simulink model "wlan_phy_rx_pmd"  (Simulink 7.8)
settings: Solver=VariableStepDiscrete  SolverMode=SingleTasking  StartTime=0.0  StopTime=rx_sim.sim_time  RelTol=1e-3
PostLoadFcn:
  | wlan_phy_rx_init
InitFcn:
  | wlan_phy_rx_init
StartFcn:
  | wlan_phy_rx_init
values from neighbouring files (symbols this model uses):
  barker_seq20 = 0.95 * circshift(barker_seq20, [0 4]) ./ max(abs(barker_seq20))    [wlan_phy_rx_init.m]
  bit_scrambler_lfsr_bytes = sum(bit_scrambler_lfsr_bytes .* repmat(2.^[0:7], 127, 1),2)    [wlan_phy_rx_init.m]
  longCorr_coef_i = [3*real(longCorr_coef)]    [wlan_phy_rx_init.m]
  longCorr_coef_q = [3*imag(longCorr_coef)]    [wlan_phy_rx_init.m]
  MAX_CP_LEN = 32    [wlan_phy_rx_init.m]
  MAX_NCBPS = NCBPS_11n_64QAM    (= 312)    [wlan_phy_rx_init.m]
  MAX_NUM_BYTES = 2^16-1    (= 65535)    [wlan_phy_rx_init.m]
  MAX_NUM_SC = 64    [wlan_phy_rx_init.m]
  MAX_NUM_SYMS = 600    [wlan_phy_rx_init.m]
  NDBPS_11a_BPSK12 = 24    [wlan_phy_rx_init.m]
  preFFT_sampBuff_numSamps = 4*MAX_NUM_SC    (= 256)    [wlan_phy_rx_init.m]
  rx_sim = struct()    [wlan_phy_rx_init.m]
  scr_ind_rev = zeros(1,128)    [wlan_phy_rx_init.m]
undefined symbols (not defined in the file or in its folder): gcbh, mcs_rom_11ag, mcs_rom_11n, wlan_rx_deinterleave_rom_gen, xlBlockLoadCallback, xlGetNormalizedPeriod, xlipgetpartsetting
libraries: xbsIndex_r4 x4943, xbsEDKLib_r4 x1
(part 6 of 28)

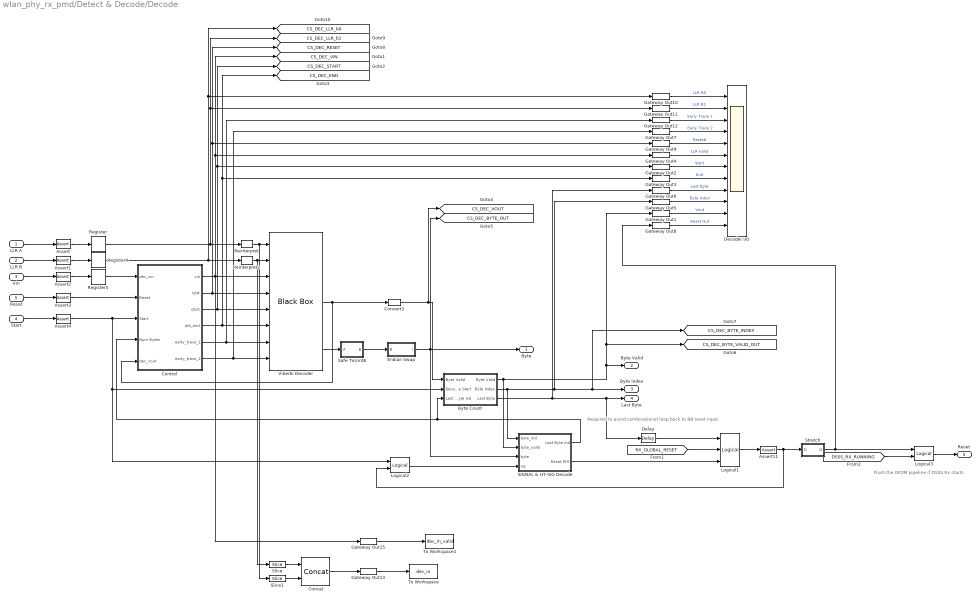
== system wlan_phy_rx_pmd/Detect & Decode/Decode ==
  note: Required to avoid combinational loop
      | back to BB reset input
  note: Flush the OFDM pipeline if DSSS Rx starts
  LLR A : Inport
  LLR B : Inport  Port=2
  Vin : Inport  Port=3
  Start : Inport  Port=4
  Reset : Inport  Port=5
  Assert{,1..4,11} (6 alike) : xbsIndex_r4/Assert  assert_type=off  type_source=Explicitly  gui_display_data_type=Fixed-point  arith_type="Signed  (2's comp)"  n_bits=16  bin_pt=14  preci_type=Single  exp_width=8  frac_width=24  assert_rate=on  rate_source=Explicitly  period=1  output_port=on  block_type=assert
      Assert11 settings: block_version=VER_STRING_GOES_HERE
  Byte Count : Subsystem
  Concat : xbsIndex_r4/Concat  num_inputs=2  dbl_ovrd=off  block_type=concat
  Control : Subsystem
  Convert3 : xbsIndex_r4/Convert  gui_display_data_type=Boolean  arith_type=Boolean  n_bits=1  bin_pt=0  float_type=Single  exp_bits=8  fraction_bits=24  quantization=Truncate  overflow=Wrap  en=off  latency=0  dbl_ovrd=off  pipeline=off  block_type=convert
  Decoder I/O : Scope  NumInputPorts=12
      settings: TimeRange=30000  YMin=-10~-10~0~-1~0~0~0~0~0~0~0~0  YMax=10~10~1~1~1~1~1~1~1~100~1~1  SaveName=ScopeData3
  Delay : xbsIndex_r4/Delay  rst=off  infoeditControl=Selection of Reset will increase slice count due to use of real FFs and instead of SRLs  en=off  latency=1  dbl_ovrd=off  reg_retiming=off  block_type=delay
  Endian Swap : Subsystem
  Gateway Out{1..13,15} (14 alike) : xbsIndex_r4/Gateway Out  inherit_from_input=off  hdl_port=off  timing_constraint=None  locs_specified=off  LOCs={}  UseAsDAC=off  DACChannel='1'  block_type=gatewayout
  Logical1 : xbsIndex_r4/Logical  logical_function=OR  inputs=3  en=off  latency=0  precision=Full  arith_type=Unsigned  n_bits=16  bin_pt=0  align_bp=on  dbl_ovrd=off  block_type=logical
  Logical2 : xbsIndex_r4/Logical  logical_function=OR  inputs=2  en=off  latency=0  precision=Full  arith_type=Unsigned  n_bits=16  bin_pt=0  align_bp=on  dbl_ovrd=off  block_type=logical
  Logical3 : xbsIndex_r4/Logical  logical_function=OR  inputs=2  en=off  latency=0  precision=Full  arith_type=Unsigned  n_bits=16  bin_pt=0  align_bp=on  dbl_ovrd=off  block_type=logical
  Register : xbsIndex_r4/Register  init=0  rst=off  en=off  dbl_ovrd=off  block_type=register
  Register4 : xbsIndex_r4/Register  init=0  rst=off  en=off  dbl_ovrd=off  block_type=register
  Register5 : xbsIndex_r4/Register  init=0  rst=off  en=off  dbl_ovrd=off  block_type=register
  Reinterpret : xbsIndex_r4/Reinterpret  force_arith_type=on  arith_type=Unsigned  force_bin_pt=on  bin_pt=0  block_type=reinterpret
  Reinterpret2 : xbsIndex_r4/Reinterpret  force_arith_type=on  arith_type=Unsigned  force_bin_pt=on  bin_pt=0  block_type=reinterpret
  SIGNAL & HT-SIG Decode : Subsystem
  Safe ToUint8 : Subsystem
  Slice : xbsIndex_r4/Slice  nbits=1  boolean_output=off  mode=Upper Bit Location + Width  bit1=0  base1=MSB of Input  bit0=0  base0=LSB of Input  dbl_ovrd=off  block_type=slice
  Slice1 : xbsIndex_r4/Slice  nbits=1  boolean_output=off  mode=Upper Bit Location + Width  bit1=0  base1=MSB of Input  bit0=0  base0=LSB of Input  dbl_ovrd=off  block_type=slice
  Stretch : Subsystem
  To Workspace : ToWorkspace  VariableName=dec_in  SampleTime=-1  SaveFormat=Array
  To Workspace1 : ToWorkspace  VariableName=dec_in_valid  SampleTime=-1  SaveFormat=Array
  Viberbi Decoder : xbsIndex_r4/Black Box  init_code=vb_decoder_top_config  sim_method=ISE Simulator  verbose=off  block_type=blackbox2
  Byte : Outport
  Byte Valid : Outport  Port=2
  Byte Index : Outport  Port=3
  Last Byte : Outport  Port=4
  " Reset" : Outport  Port=5
  tag scope: CS_DEC_BYTE_INDEX=global, CS_DEC_BYTE_OUT=global, CS_DEC_BYTE_VALID_OUT=global, CS_DEC_END=global, CS_DEC_LLR_b0=global, CS_DEC_LLR_b1=global, CS_DEC_RESET=global, CS_DEC_START=global, CS_DEC_VIN=global, CS_DEC_VOUT=global
  wires:
  Register/1 -> Reinterpret/1, Gateway Out11/1, [CS_DEC_LLR_b1]
  Register4/1 -> Reinterpret2/1, Gateway Out10/1, [CS_DEC_LLR_b0]
  Register5/1 -> Control/1(dec_vin)
  Viberbi Decoder/2 -> Convert3/1, Control/5(dec_vout)
  Viberbi Decoder/3 -> Safe ToUint8/1(A)
  Start/1 -> Assert4/1
  Safe ToUint8/1(B) -> Endian Swap/1(B)
  Byte Count/2(Byte Index) -> Byte Index/1, [CS_DEC_BYTE_INDEX], Gateway Out5/1, SIGNAL & HT-SIG Decode/1(byte_ind)
  Gateway Out1/1 -> Decoder I/O/11  "Vout"
  Gateway Out5/1 -> Decoder I/O/10  "Byte Index"
  Gateway Out6/1 -> Decoder I/O/9  "Last Byte"
  Gateway Out4/1 -> Decoder I/O/6  "LLR Valid"
  Gateway Out2/1 -> Decoder I/O/7  "Start"
  Gateway Out3/1 -> Decoder I/O/8  "End"
  Assert11/1 -> Logical2/2, Stretch/1(D)
  Logical1/1 -> Assert11/1
  Gateway Out8/1 -> Decoder I/O/12  "Reset Out"
  Logical2/1 -> SIGNAL & HT-SIG Decode/4(rst)
  Vin/1 -> Assert2/1
  LLR B/1 -> Assert1/1
  LLR A/1 -> Assert/1
  Byte Count/3(Last Byte) -> Last Byte/1, Delay/1, Gateway Out6/1
  SIGNAL & HT-SIG Decode/1(Last Byte Ind) -> Byte Count/3(Last Byte Ind), Control/4(Num Bytes)
  Byte Count/1(" Byte Valid") -> Byte Valid/1, [CS_DEC_BYTE_VALID_OUT], Gateway Out1/1, SIGNAL & HT-SIG Decode/2(byte_valid)
  Stretch/1(Q) -> Gateway Out8/1, Logical3/1
  Convert3/1 -> Byte Count/1(Byte Valid), [CS_DEC_VOUT]
  Gateway Out9/1 -> Decoder I/O/5  "Reset#"
  Delay/1 -> Logical1/1
  Gateway Out10/1 -> Decoder I/O/1  "LLR B0"
  Gateway Out11/1 -> Decoder I/O/2  "LLR B1"
  Gateway Out12/1 -> Decoder I/O/3  "Early Trace 1"
  Control/1(vin) -> Viberbi Decoder/3, Gateway Out4/1, [CS_DEC_VIN], Gateway Out15/1
  Control/2(rst#) -> Viberbi Decoder/4, Gateway Out9/1, [CS_DEC_RESET]
  Control/3(start) -> Viberbi Decoder/5, Gateway Out2/1, [CS_DEC_START]
  Control/4(pkt_end) -> Viberbi Decoder/6, Gateway Out3/1, [CS_DEC_END]
  Control/5(early_trace_1) -> Viberbi Decoder/7, Gateway Out12/1
  Reset/1 -> Assert3/1
  Reinterpret/1 -> Viberbi Decoder/1, Slice1/1
  Reinterpret2/1 -> Viberbi Decoder/2, Slice/1
  Control/6(early_trace_2) -> Viberbi Decoder/8, Gateway Out7/1
  Gateway Out7/1 -> Decoder I/O/4  "Early Trace 2"
  Endian Swap/1(I) -> Byte/1, [CS_DEC_BYTE_OUT], SIGNAL & HT-SIG Decode/3(byte)
  [RX_GLOBAL_RESET] -> Logical1/2
  SIGNAL & HT-SIG Decode/2(Reset PHY) -> Logical1/3
  Assert/1 -> Register/1
  Assert1/1 -> Register4/1
  Assert2/1 -> Register5/1
  Assert3/1 -> Control/2(Reset)
  Assert4/1 -> Byte Count/2(Decode Start), Logical2/1, Control/3(Start)
  [DSSS_RX_RUNNING] -> Logical3/2
  Logical3/1 -> " Reset"/1
  Gateway Out15/1 -> To Workspace1/1
  Concat/1 -> Gateway Out13/1
  Gateway Out13/1 -> To Workspace/1
  Slice/1 -> Concat/1
  Slice1/1 -> Concat/2

== system wlan_phy_rx_pmd/Detect & Decode/Descramble ==
  note: SERVICE field starts after
      | SIGNAL fields, byte 3 for 11a/g
      | byte 9 for 11n/ac
  Byte : Inport
  Byte Valid : Inport  Port=2
  Byte Index : Inport  Port=3
  Last Byte : Inport  Port=4
  Constant : xbsIndex_r4/Constant  const=3  gui_display_data_type=Fixed-point  arith_type=Unsigned  n_bits=4  bin_pt=0  preci_type=Single  exp_width=8  frac_width=24  explicit_period=off  period=1  equ=P=C  opselect=C  inp2=PCIN>>17  opr=+  inp1=P  carry=CIN  dbl_ovrd=off  block_type=constant
  Constant2 : xbsIndex_r4/Constant  const=9  gui_display_data_type=Fixed-point  arith_type=Unsigned  n_bits=4  bin_pt=0  preci_type=Single  exp_width=8  frac_width=24  explicit_period=off  period=1  equ=P=C  opselect=C  inp2=PCIN>>17  opr=+  inp1=P  carry=CIN  dbl_ovrd=off  block_type=constant
  DOUT : xbsIndex_r4/Register  init=0  rst=off  en=on  dbl_ovrd=off  block_type=register
  Delay{,1,3..4} (4 alike) : xbsIndex_r4/Delay  rst=off  infoeditControl=Selection of Reset will increase slice count due to use of real FFs and instead of SRLs  en=off  latency=3  dbl_ovrd=off  reg_retiming=off  block_type=delay
  Descram : Scope  NumInputPorts=6
      settings: TimeRange=10000  YMin=0~0~32.5~-1~-1~0  YMax=1~275~52.5~1~1~250  SaveName=ScopeData18
  Descram Byte Calc : Subsystem
  Gateway Out{1..9} (9 alike) : xbsIndex_r4/Gateway Out  inherit_from_input=off  hdl_port=off  timing_constraint=None  locs_specified=off  LOCs={}  UseAsDAC=off  DACChannel='1'  block_type=gatewayout
  Logical1 : xbsIndex_r4/Logical  logical_function=XOR  inputs=2  en=off  latency=0  precision=Full  arith_type=Unsigned  n_bits=16  bin_pt=0  align_bp=on  dbl_ovrd=off  block_type=logical
  Mux : xbsIndex_r4/Mux  inputs=2  en=off  latency=0  precision=Full  arith_type=Unsigned  n_bits=16  bin_pt=14  quantization=Truncate  overflow=Wrap  dbl_ovrd=off  block_type=mux
  To Workspace : ToWorkspace  VariableName=byteout  SampleTime=-1  SaveFormat=Array
  To Workspace1 : ToWorkspace  VariableName=byteout_valid  SampleTime=-1  SaveFormat=Array
  To Workspace2 : ToWorkspace  VariableName=byteout_index  SampleTime=-1  SaveFormat=Array
  " Byte" : Outport
  " Byte Valid" : Outport  Port=2
  " Byte Index" : Outport  Port=3
  " Last Byte" : Outport  Port=4
  wires:
  Gateway Out4/1 -> Descram/6  "Registered Output Byte"
  DOUT/1 -> Gateway Out4/1
  Delay/1 -> Logical1/1, Gateway Out9/1
  Gateway Out1/1 -> Descram/3  "Byte Index"
  Gateway Out6/1 -> Descram/2  "Byte Out"
  Gateway Out5/1 -> Descram/1  "Byte Valid"
  Delay4/1 -> Gateway Out1/1, " Byte Index"/1, Gateway Out7/1
  Delay3/1 -> Gateway Out5/1, " Byte Valid"/1, DOUT/2, Gateway Out3/1
  Logical1/1 -> " Byte"/1, Gateway Out6/1, DOUT/1, Gateway Out2/1
  Descram Byte Calc/1(Descram Val) -> Logical1/2, Gateway Out8/1
  Byte/1 -> Descram Byte Calc/1(data_byte), Delay/1
  Byte Index/1 -> Descram Byte Calc/3(data_ind), Delay4/1
  Byte Valid/1 -> Descram Byte Calc/2(data_valid), Delay3/1
  Last Byte/1 -> Delay1/1
  Delay1/1 -> " Last Byte"/1
  Gateway Out3/1 -> To Workspace1/1
  Gateway Out2/1 -> To Workspace/1
  Gateway Out7/1 -> To Workspace2/1
  Gateway Out8/1 -> Descram/4  "Descram Byte"
  Gateway Out9/1 -> Descram/5  "Input Byte"
  [RX_PHY_MODE_11N_AC] -> Mux/1
  Mux/1 -> Descram Byte Calc/4(Svc byte ind)
  Constant/1 -> Mux/2
  Constant2/1 -> Mux/3

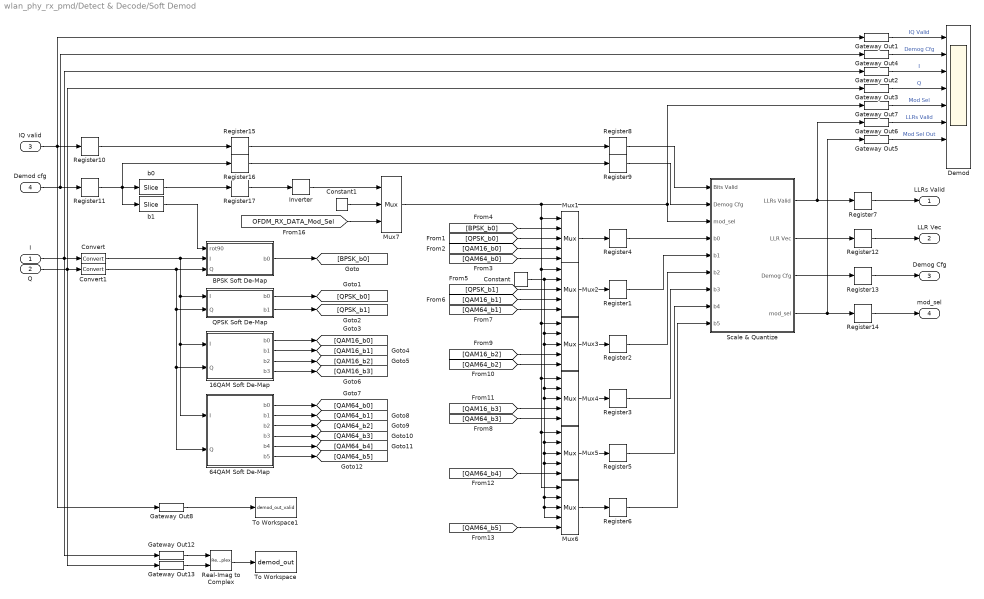
== system wlan_phy_rx_pmd/Detect & Decode/Soft Demod ==
  I : Inport
  Q : Inport  Port=2
  IQ valid : Inport  Port=3
  Demod cfg : Inport  Port=4
  16QAM Soft De-Map : Subsystem
  64QAM Soft De-Map : Subsystem
  BPSK Soft De-Map : Subsystem
  Constant : xbsIndex_r4/Constant  const=0  gui_display_data_type=Fixed-point  arith_type=Signed (2's comp)  n_bits=9  bin_pt=7  preci_type=Single  exp_width=8  frac_width=24  explicit_period=off  period=1  equ=P=C  opselect=C  inp2=PCIN>>17  opr=+  inp1=P  carry=CIN  dbl_ovrd=off  block_type=constant
  Constant1 : xbsIndex_r4/Constant  const=0  gui_display_data_type=Fixed-point  arith_type=Unsigned  n_bits=2  bin_pt=0  preci_type=Single  exp_width=8  frac_width=24  explicit_period=off  period=1  equ=P=C  opselect=C  inp2=PCIN>>17  opr=+  inp1=P  carry=CIN  dbl_ovrd=off  block_type=constant
      settings: block_version=VER_STRING_GOES_HERE
  Convert : xbsIndex_r4/Convert  gui_display_data_type=Fixed-point  arith_type="Signed  (2's comp)"  n_bits=18  bin_pt=16  float_type=Single  exp_bits=8  fraction_bits=24  quantization=Truncate  overflow=Saturate  en=off  latency=1  dbl_ovrd=off  pipeline=off  block_type=convert
  Convert1 : xbsIndex_r4/Convert  gui_display_data_type=Fixed-point  arith_type="Signed  (2's comp)"  n_bits=18  bin_pt=16  float_type=Single  exp_bits=8  fraction_bits=24  quantization=Truncate  overflow=Saturate  en=off  latency=1  dbl_ovrd=off  pipeline=off  block_type=convert
  Demod : Scope  NumInputPorts=8
      settings: TimeRange=9000  YMin=-0.1~0~0~0~-1~-0.1~41.76470588235293~-0.1  YMax=1.1~1~1~1000~1~1.1~60.58823529411768~1.1  SaveName=ScopeData46
  Gateway Out{1..8,12..13} (10 alike) : xbsIndex_r4/Gateway Out  inherit_from_input=off  hdl_port=off  timing_constraint=None  locs_specified=off  LOCs={}  UseAsDAC=off  DACChannel='1'  block_type=gatewayout
  Inverter : xbsIndex_r4/Inverter  en=off  latency=0  dbl_ovrd=off  block_type=inv
  Mux{1..6} (6 alike) : xbsIndex_r4/Mux  inputs=4  en=off  latency=0  precision=Full  arith_type=Unsigned  n_bits=16  bin_pt=14  quantization=Truncate  overflow=Wrap  dbl_ovrd=off  block_type=mux
  Mux7 : xbsIndex_r4/Mux  inputs=2  en=off  latency=1  precision=Full  arith_type=Unsigned  n_bits=16  bin_pt=14  quantization=Truncate  overflow=Wrap  dbl_ovrd=off  block_type=mux
  QPSK Soft De-Map : Subsystem
  Real-Imag to Complex : RealImagToComplex
  Register{1..17} (17 alike) : xbsIndex_r4/Register  init=0  rst=off  en=off  dbl_ovrd=off  block_type=register
  Scale & Quantize : Subsystem
  To Workspace : ToWorkspace  VariableName=demod_out  SampleTime=-1  SaveFormat=Array
  To Workspace1 : ToWorkspace  VariableName=demod_out_valid  SampleTime=-1  SaveFormat=Array
  b0 : xbsIndex_r4/Slice  nbits=1  boolean_output=on  mode=Lower Bit Location + Width  bit1=0  base1=MSB of Input  bit0=0  base0=LSB of Input  dbl_ovrd=off  block_type=slice
  b1 : xbsIndex_r4/Slice  nbits=1  boolean_output=on  mode=Lower Bit Location + Width  bit1=0  base1=MSB of Input  bit0=1  base0=LSB of Input  dbl_ovrd=off  block_type=slice
  LLRs Valid : Outport
  LLR Vec : Outport  Port=2
  " Demog Cfg" : Outport  Port=3
  " mod_sel" : Outport  Port=4
  wires:
  64QAM Soft De-Map/1(b0) -> [QAM64_b0]
  64QAM Soft De-Map/2(b1) -> [QAM64_b1]
  64QAM Soft De-Map/3(b2) -> [QAM64_b2]
  64QAM Soft De-Map/4(b3) -> [QAM64_b3]
  64QAM Soft De-Map/5(b4) -> [QAM64_b4]
  64QAM Soft De-Map/6(b5) -> [QAM64_b5]
  16QAM Soft De-Map/1(b0) -> [QAM16_b0]
  16QAM Soft De-Map/2(b1) -> [QAM16_b1]
  16QAM Soft De-Map/3(b2) -> [QAM16_b2]
  16QAM Soft De-Map/4(b3) -> [QAM16_b3]
  QPSK Soft De-Map/1(b0) -> [QPSK_b0]
  QPSK Soft De-Map/2(b1) -> [QPSK_b1]
  BPSK Soft De-Map/1(b0) -> [BPSK_b0]
  Mux7/1 -> Mux1/1, Mux2/1, Mux3/1, Mux4/1, Mux5/1, Mux6/1, Scale & Quantize/3(mod_sel), Gateway Out7/1
  Constant/1 -> Mux2/2, Mux3/2, Mux3/3, Mux4/2, Mux4/3, Mux5/2, Mux5/3, Mux5/4, Mux6/2, Mux6/3, Mux6/4
  [BPSK_b0] -> Mux1/2
  [QPSK_b0] -> Mux1/3
  [QAM16_b0] -> Mux1/4
  [QAM64_b0] -> Mux1/5
  [QPSK_b1] -> Mux2/3
  [QAM16_b1] -> Mux2/4
  [QAM64_b1] -> Mux2/5
  [QAM16_b2] -> Mux3/4
  [QAM64_b2] -> Mux3/5
  [QAM16_b3] -> Mux4/4
  [QAM64_b3] -> Mux4/5
  [QAM64_b4] -> Mux5/5
  [QAM64_b5] -> Mux6/5
  Mux1/1 -> Register4/1
  Mux2/1 -> Register1/1
  Mux3/1 -> Register2/1
  Mux4/1 -> Register3/1
  Mux5/1 -> Register5/1
  Mux6/1 -> Register6/1
  I/1 -> Convert/1, Gateway Out2/1, Gateway Out12/1
  Q/1 -> Convert1/1, Gateway Out3/1, Gateway Out13/1
  b0/1 -> Register17/1
  Demod cfg/1 -> Register11/1, Gateway Out4/1
  b1/1 -> BPSK Soft De-Map/1(rot90)
  [OFDM_RX_DATA_Mod_Sel] -> Mux7/3
  Constant1/1 -> Mux7/2
  Inverter/1 -> Mux7/1
  IQ valid/1 -> Register10/1, Gateway Out1/1, Gateway Out8/1
  Register8/1 -> Scale & Quantize/1(Bits Valid)
  Register4/1 -> Scale & Quantize/4(b0)
  Register1/1 -> Scale & Quantize/5(b1)
  Register2/1 -> Scale & Quantize/6(b2)
  Register3/1 -> Scale & Quantize/7(b3)
  Register5/1 -> Scale & Quantize/8(b4)
  Register6/1 -> Scale & Quantize/9(b5)
  Register9/1 -> Scale & Quantize/2(Demog Cfg)
  Scale & Quantize/1(LLRs Valid) -> Gateway Out6/1, Register7/1
  Scale & Quantize/2(LLR Vec) -> Register12/1
  Scale & Quantize/3(" Demog Cfg") -> Register13/1
  Scale & Quantize/4(" mod_sel") -> Gateway Out5/1, Register14/1
  Convert/1 -> BPSK Soft De-Map/2(I), QPSK Soft De-Map/1(I), 16QAM Soft De-Map/1(I), 64QAM Soft De-Map/1(I)
  Convert1/1 -> BPSK Soft De-Map/3(Q), QPSK Soft De-Map/2(Q), 16QAM Soft De-Map/2(Q), 64QAM Soft De-Map/2(Q)
  Register10/1 -> Register15/1
  Register11/1 -> b1/1, b0/1, Register16/1
  Gateway Out4/1 -> Demod/2  "Demog Cfg"
  Gateway Out1/1 -> Demod/1  "IQ Valid"
  Gateway Out2/1 -> Demod/3  "I"
  Gateway Out3/1 -> Demod/4  "Q"
  Gateway Out5/1 -> Demod/7  "Mod Sel Out"
  Gateway Out6/1 -> Demod/6  "LLRs Valid"
  Gateway Out12/1 -> Real-Imag to Complex/1
  Gateway Out13/1 -> Real-Imag to Complex/2
  Real-Imag to Complex/1 -> To Workspace/1
  Gateway Out8/1 -> To Workspace1/1
  Register7/1 -> LLRs Valid/1
  Register12/1 -> LLR Vec/1
  Register13/1 -> " Demog Cfg"/1
  Register14/1 -> " mod_sel"/1
  Gateway Out7/1 -> Demod/5  "Mod Sel"
  Register15/1 -> Register8/1
  Register16/1 -> Register9/1
  Register17/1 -> Inverter/1

== system wlan_phy_rx_pmd/Detect & Decode/Subcarrier Map & Demod Control ==
  note: Syms 5 and 6 have no data in 11n/ac
      | (HT STF, HTLTF)
  note: OFDM Symbols in 11a/g:
      | 0: LTF (BPSK)
      | 1: LTF (BPSK)
      | 2: SIG (BPSK) 48sc
      | 3-N: Data  48sc
  note: OFDM Symbols in 11n SISO:
      | 0: L-LTF (BPSK)
      | 1: L-LTF (BPSK)
      | 2: L-SIG (BPSK) 48sc
      | 3: HT-SIG1 (QBPSK) 48sc
      | 4: HT-SIG2 (QBPSK) 48sc
      | 5: HT-STF
      | 6: HT-LFT1
      | 7-N: Data 52sc
  note: OFDM Symbols in 11ac SISO:
      | 0: L-LTF (BPSK)
      | 1: L-LTF (BPSK)
      | 2: L-SIG (BPSK) 48sc
      | 3: VHT-SIGA1 (BPSK) 48sc
      | 4: VHT-SIGA2 (QBPSK) 48sc
      | 5: VHT-STF
      | 6: VHT-LFT1
      | 7: VHT-SIGB 52sc
      | 8-N: Data 52sc
  note: Lookup tables below are 0 latency - add delays to I/Q here
      | if that changes in a future rev
  note: Capture current PHY mode decision
      | at start of each OFDM sym (subcarrier 0)
      | Every PHY mode sets subcarrier 0 to 0,
      | so one cycle ambiguity about PHY mode
      | at subcarrier 0 is no problem
  Eq I : Inport
  Eq Q : Inport  Port=2
  iq_valid : Inport  Port=3
  sc_ind : Inport  Port=4
  Sym ind : Inport  Port=5
  Reset : Inport  Port=6
  Concat : xbsIndex_r4/Concat  num_inputs=2  dbl_ovrd=off  block_type=concat
  Constant1 : xbsIndex_r4/Constant  const=0  gui_display_data_type=Fixed-point  arith_type=Unsigned  n_bits=1  bin_pt=0  preci_type=Single  exp_width=8  frac_width=24  explicit_period=off  period=1  equ=P=C  opselect=C  inp2=PCIN>>17  opr=+  inp1=P  carry=CIN  dbl_ovrd=off  block_type=constant
  Constant2 : xbsIndex_r4/Constant  const=1  gui_display_data_type=Fixed-point  arith_type=Unsigned  n_bits=2  bin_pt=0  preci_type=Single  exp_width=8  frac_width=24  explicit_period=off  period=1  equ=P=C  opselect=C  inp2=PCIN>>17  opr=+  inp1=P  carry=CIN  dbl_ovrd=off  block_type=constant
  Constant3 : xbsIndex_r4/Constant  const=5  gui_display_data_type=Fixed-point  arith_type=Unsigned  n_bits=3  bin_pt=0  preci_type=Single  exp_width=8  frac_width=24  explicit_period=off  period=1  equ=P=C  opselect=C  inp2=PCIN>>17  opr=+  inp1=P  carry=CIN  dbl_ovrd=off  block_type=constant
  Constant4 : xbsIndex_r4/Constant  const=6  gui_display_data_type=Fixed-point  arith_type=Unsigned  n_bits=3  bin_pt=0  preci_type=Single  exp_width=8  frac_width=24  explicit_period=off  period=1  equ=P=C  opselect=C  inp2=PCIN>>17  opr=+  inp1=P  carry=CIN  dbl_ovrd=off  block_type=constant
  Constant5 : xbsIndex_r4/Constant  const=2  gui_display_data_type=Fixed-point  arith_type=Unsigned  n_bits=2  bin_pt=0  preci_type=Single  exp_width=8  frac_width=24  explicit_period=off  period=1  equ=P=C  opselect=C  inp2=PCIN>>17  opr=+  inp1=P  carry=CIN  dbl_ovrd=off  block_type=constant
  Convert : xbsIndex_r4/Convert  gui_display_data_type=Boolean  arith_type=Boolean  n_bits=16  bin_pt=14  float_type=Single  exp_bits=8  fraction_bits=24  quantization=Truncate  overflow=Wrap  en=off  latency=0  dbl_ovrd=off  pipeline=off  block_type=convert
  Demod Config : Subsystem
  Logical : xbsIndex_r4/Logical  logical_function=AND  inputs=3  en=off  latency=0  precision=Full  arith_type=Unsigned  n_bits=16  bin_pt=0  align_bp=on  dbl_ovrd=off  block_type=logical
  Logical1 : xbsIndex_r4/Logical  logical_function=OR  inputs=2  en=off  latency=0  precision=Full  arith_type=Unsigned  n_bits=16  bin_pt=0  align_bp=on  dbl_ovrd=off  block_type=logical
  Logical2 : xbsIndex_r4/Logical  logical_function=NAND  inputs=2  en=off  latency=0  precision=Full  arith_type=Unsigned  n_bits=16  bin_pt=0  align_bp=on  dbl_ovrd=off  block_type=logical
  Logical3 : xbsIndex_r4/Logical  logical_function=AND  inputs=2  en=off  latency=0  precision=Full  arith_type=Unsigned  n_bits=16  bin_pt=0  align_bp=on  dbl_ovrd=off  block_type=logical
  Logical4 : xbsIndex_r4/Logical  logical_function=OR  inputs=2  en=off  latency=0  precision=Full  arith_type=Unsigned  n_bits=8  bin_pt=2  align_bp=on  dbl_ovrd=off  block_type=logical
      settings: xl_area=[1 0 0 1 0 0 0]  block_version=VER_STRING_GOES_HERE
  Mux : xbsIndex_r4/Mux  inputs=2  en=off  latency=0  precision=Full  arith_type=Unsigned  n_bits=16  bin_pt=14  quantization=Truncate  overflow=Wrap  dbl_ovrd=off  block_type=mux
  Posedge : Subsystem
  Posedge1 : Subsystem
  Register : xbsIndex_r4/Register  init=0  rst=off  en=on  dbl_ovrd=off  block_type=register
  Relational1 : xbsIndex_r4/Relational  mode=a>b  en=off  latency=0  dbl_ovrd=off  block_type=relational
  Relational2 : xbsIndex_r4/Relational  mode=a=b  en=off  latency=0  dbl_ovrd=off  block_type=relational
  Relational3 : xbsIndex_r4/Relational  mode=a=b  en=off  latency=0  dbl_ovrd=off  block_type=relational
  Relational4 : xbsIndex_r4/Relational  mode=a=b  en=off  latency=0  dbl_ovrd=off  block_type=relational
  Relational5 : xbsIndex_r4/Relational  mode=a>b  en=off  latency=0  dbl_ovrd=off  block_type=relational
  Relational6 : xbsIndex_r4/Relational  mode=a>b  en=off  latency=0  dbl_ovrd=off  block_type=relational
  SC Sym Map 11a : xbsIndex_r4/ROM  depth=MAX_NUM_SC  initVector=sc_data_sym_map_11a_bool  distributed_mem=Distributed memory  rst=off  init_reg=0  en=off  latency=0  gui_display_data_type=Boolean  arith_type=Boolean  n_bits=ceil(log2(MAX_NUM_SC))+1  bin_pt=0  preci_type=Single  exp_width=8  frac_width=24  dbl_ovrd=off  optimize=Area  use_rpm=on  block_type=sprom
  SC Sym Map 11n/ac : xbsIndex_r4/ROM  depth=MAX_NUM_SC  initVector=sc_data_sym_map_11n_bool  distributed_mem=Distributed memory  rst=off  init_reg=0  en=off  latency=0  gui_display_data_type=Boolean  arith_type=Boolean  n_bits=ceil(log2(MAX_NUM_SC))+1  bin_pt=0  preci_type=Single  exp_width=8  frac_width=24  dbl_ovrd=off  optimize=Area  use_rpm=on  block_type=sprom
  Data I : Outport
  Data Q : Outport  Port=2
  data_sc : Outport  Port=3
  Demod cfg : Outport  Port=4
  Reset Demod : Outport  Port=5
  Start Decoder : Outport  Port=6
  wires:
  sc_ind/1 -> SC Sym Map 11a/1, SC Sym Map 11n/ac/1, Demod Config/1(sc_ind), Relational2/2
  SC Sym Map 11a/1 -> Mux/2
  iq_valid/1 -> Convert/1
  Convert/1 -> Logical/3
  Mux/1 -> Logical/2
  SC Sym Map 11n/ac/1 -> Mux/3
  Register/1 -> Logical2/2, Logical3/1
  Logical/1 -> data_sc/1
  Constant3/1 -> Relational3/1
  Constant4/1 -> Relational4/1, Relational1/2
  Sym ind/1 -> Relational3/2, Relational4/2, Relational1/1, Relational5/1, Relational6/1, Demod Config/2(sym_ind)
  Relational3/1 -> Logical1/1
  Relational4/1 -> Logical1/2
  Logical1/1 -> Logical2/1
  Logical2/1 -> Logical/1
  Logical3/1 -> Mux/1
  Relational1/1 -> Logical3/2
  Eq I/1 -> Data I/1
  Eq Q/1 -> Data Q/1
  [RX_PHY_MODE_11N_AC] -> Register/1
  Constant1/1 -> Relational2/1
  Relational2/1 -> Register/2
  Constant2/1 -> Relational6/2
  Relational6/1 -> Posedge1/1(D)
  Constant5/1 -> Relational5/2
  Relational5/1 -> Posedge/1(D)
  Posedge1/1(Q) -> Logical4/2
  Posedge/1(Q) -> Start Decoder/1
  Logical4/1 -> Reset Demod/1
  Reset/1 -> Logical4/1
  Concat/1 -> Demod cfg/1
  Demod Config/2(QBPSK Sym) -> Concat/1
  Demod Config/3(SIGNAL Sym) -> Concat/2

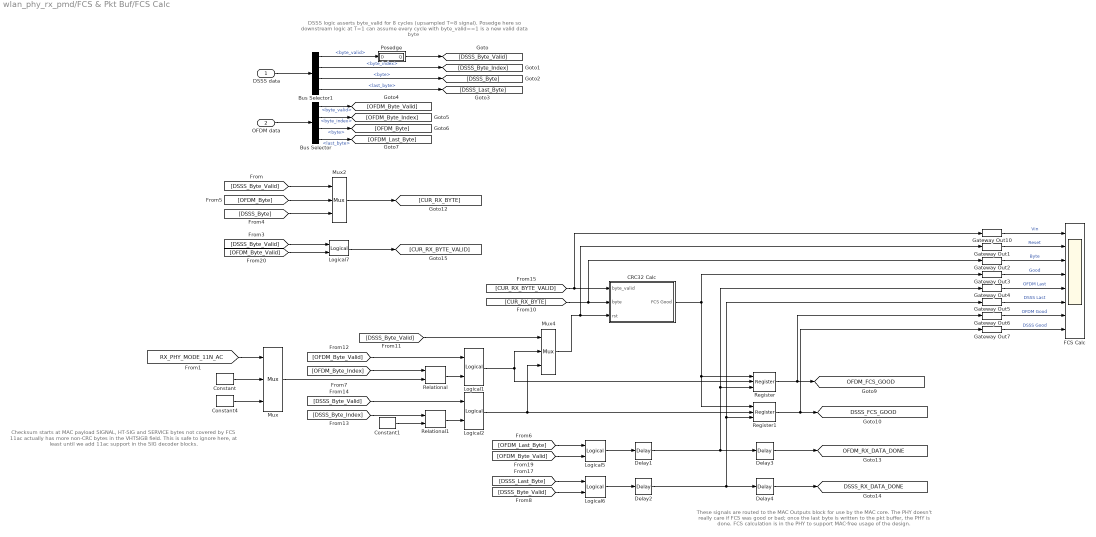
== system wlan_phy_rx_pmd/FCS & Pkt Buf/FCS Calc ==
  note: These signals are routed to the MAC Outputs
      | block for use by the MAC core. The PHY doesn't
      | really care if FCS was good or bad; once the
      | last byte is written to the pkt buffer, the PHY is done.
      | FCS calculation is in the PHY to support MAC-free
      | usage of the design.
  note: DSSS logic asserts byte_valid for 8 cycles (upsampled T=8 signal).
      | Posedge here so downstream logic at T=1 can assume every cycle
      | with byte_valid==1 is a new valid data byte
  note: Checksum starts at MAC payload
      | SIGNAL, HT-SIG and SERVICE
      | bytes not covered by FCS
      |
      | 11ac actually has more non-CRC bytes
      | in the VHTSIGB field. This is safe to
      | ignore here, at least until we add 11ac
      | support in the SIG decoder blocks.
  DSSS data : Inport
  OFDM data : Inport  Port=2
  Bus Selector : BusSelector  OutputSignals=byte_valid,byte_index,byte,last_byte
  Bus Selector1 : BusSelector  OutputSignals=byte_valid,byte_index,byte,last_byte
  CRC32 Calc : Subsystem
  Constant : xbsIndex_r4/Constant  const=4  gui_display_data_type=Fixed-point  arith_type=Unsigned  n_bits=3  bin_pt=0  preci_type=Single  exp_width=8  frac_width=24  explicit_period=off  period=1  equ=P=C  opselect=C  inp2=PCIN>>17  opr=+  inp1=P  carry=CIN  dbl_ovrd=off  block_type=constant
  Constant1 : xbsIndex_r4/Constant  const=5  gui_display_data_type=Fixed-point  arith_type=Unsigned  n_bits=3  bin_pt=0  preci_type=Single  exp_width=8  frac_width=24  explicit_period=off  period=1  equ=P=C  opselect=C  inp2=PCIN>>17  opr=+  inp1=P  carry=CIN  dbl_ovrd=off  block_type=constant
  Constant4 : xbsIndex_r4/Constant  const=10  gui_display_data_type=Fixed-point  arith_type=Unsigned  n_bits=4  bin_pt=0  preci_type=Single  exp_width=8  frac_width=24  explicit_period=off  period=1  equ=P=C  opselect=C  inp2=PCIN>>17  opr=+  inp1=P  carry=CIN  dbl_ovrd=off  block_type=constant
  Delay{1..4} (4 alike) : xbsIndex_r4/Delay  rst=off  infoeditControl=Selection of Reset will increase slice count due to use of real FFs and instead of SRLs  en=off  latency=1  dbl_ovrd=off  reg_retiming=off  block_type=delay
  FCS Calc : Scope  NumInputPorts=8
      settings: TimeRange=16000  YMin=0~0~0~-1~0~-1~-1~-1  YMax=1~1~275~1~1~1~1~1  SaveName=ScopeData22
  Gateway Out{1..7,10} (8 alike) : xbsIndex_r4/Gateway Out  inherit_from_input=off  hdl_port=off  timing_constraint=None  locs_specified=off  LOCs={}  UseAsDAC=off  DACChannel='1'  block_type=gatewayout
  Logical{1..2,5..6} (4 alike) : xbsIndex_r4/Logical  logical_function=AND  inputs=2  en=off  latency=0  precision=Full  arith_type=Unsigned  n_bits=8  bin_pt=2  align_bp=on  dbl_ovrd=off  block_type=logical
      settings: xl_area=[0, 0, 0, 0, 0, 0, 0]  block_version=VER_STRING_GOES_HERE
  Logical7 : xbsIndex_r4/Logical  logical_function=OR  inputs=2  en=off  latency=0  precision=Full  arith_type=Unsigned  n_bits=8  bin_pt=2  align_bp=on  dbl_ovrd=off  block_type=logical
      settings: xl_area=[0, 0, 0, 0, 0, 0, 0]  block_version=VER_STRING_GOES_HERE
  Mux : xbsIndex_r4/Mux  inputs=2  en=off  latency=0  precision=Full  arith_type=Unsigned  n_bits=16  bin_pt=14  quantization=Truncate  overflow=Wrap  dbl_ovrd=off  block_type=mux
  Mux2 : xbsIndex_r4/Mux  inputs=2  en=off  latency=0  precision=Full  arith_type=Unsigned  n_bits=16  bin_pt=14  quantization=Truncate  overflow=Wrap  dbl_ovrd=off  block_type=mux
  Mux4 : xbsIndex_r4/Mux  inputs=2  en=off  latency=0  precision=Full  arith_type=Unsigned  n_bits=16  bin_pt=14  quantization=Truncate  overflow=Wrap  dbl_ovrd=off  block_type=mux
  Posedge : Subsystem
  Register : xbsIndex_r4/Register  init=0  rst=on  en=on  dbl_ovrd=off  block_type=register
  Register1 : xbsIndex_r4/Register  init=0  rst=on  en=on  dbl_ovrd=off  block_type=register
  Relational : xbsIndex_r4/Relational  mode=a<=b  en=off  latency=0  dbl_ovrd=off  block_type=relational
  Relational1 : xbsIndex_r4/Relational  mode=a<=b  en=off  latency=0  dbl_ovrd=off  block_type=relational
  tag scope: DSSS_FCS_GOOD=global, DSSS_RX_DATA_DONE=global, OFDM_FCS_GOOD=global, OFDM_RX_DATA_DONE=global
  wires:
  [DSSS_Byte_Valid] -> Mux2/1
  [OFDM_Byte] -> Mux2/2
  [DSSS_Byte] -> Mux2/3
  Mux2/1 -> [CUR_RX_BYTE]
  [DSSS_Byte_Valid] -> Logical7/1
  [OFDM_Byte_Valid] -> Logical7/2
  Logical7/1 -> [CUR_RX_BYTE_VALID]
  Mux4/1 -> CRC32 Calc/3(rst), Gateway Out1/1
  [DSSS_Byte_Valid] -> Mux4/1
  Register1/1 -> [DSSS_FCS_GOOD], Gateway Out7/1
  Register/1 -> [OFDM_FCS_GOOD], Gateway Out6/1
  CRC32 Calc/1(FCS Good) -> Register/1, Register1/1, Gateway Out3/1
  Delay2/1 -> Delay4/1, Register1/3, Gateway Out5/1
  Delay1/1 -> Delay3/1, Register/3, Gateway Out4/1
  Logical6/1 -> Delay2/1
  Logical5/1 -> Delay1/1
  [DSSS_Byte_Valid] -> Logical6/2
  [DSSS_Last_Byte] -> Logical6/1
  [OFDM_Byte_Valid] -> Logical5/2
  [OFDM_Last_Byte] -> Logical5/1
  [CUR_RX_BYTE_VALID] -> CRC32 Calc/1(byte_valid), Gateway Out10/1
  Logical2/1 -> Mux4/3, Register1/2
  [DSSS_Byte_Valid] -> Logical2/1
  Relational1/1 -> Logical2/2
  Constant1/1 -> Relational1/2
  [DSSS_Byte_Index] -> Relational1/1
  Logical1/1 -> Mux4/2, Register/2
  [OFDM_Byte_Valid] -> Logical1/1
  Relational/1 -> Logical1/2
  Mux/1 -> Relational/2
  [OFDM_Byte_Index] -> Relational/1
  [CUR_RX_BYTE] -> CRC32 Calc/2(byte), Gateway Out2/1
  Delay3/1 -> [OFDM_RX_DATA_DONE]
  Delay4/1 -> [DSSS_RX_DATA_DONE]
  Gateway Out10/1 -> FCS Calc/1  "Vin"
  Gateway Out1/1 -> FCS Calc/2  "Reset"
  Gateway Out2/1 -> FCS Calc/3  "Byte"
  Gateway Out3/1 -> FCS Calc/4  "Good"
  Gateway Out4/1 -> FCS Calc/5  "OFDM Last"
  Gateway Out5/1 -> FCS Calc/6  "DSSS Last"
  Gateway Out6/1 -> FCS Calc/7  "OFDM Good"
  Gateway Out7/1 -> FCS Calc/8  "DSSS Good"
  Bus Selector1/1 -> Posedge/1(D)  "<byte_valid>"
  Bus Selector1/2 -> [DSSS_Byte_Index]  "<byte_index>"
  Bus Selector1/3 -> [DSSS_Byte]  "<byte>"
  Bus Selector1/4 -> [DSSS_Last_Byte]  "<last_byte>"
  Bus Selector/1 -> [OFDM_Byte_Valid]  "<byte_valid>"
  Bus Selector/2 -> [OFDM_Byte_Index]  "<byte_index>"
  Bus Selector/3 -> [OFDM_Byte]  "<byte>"
  Bus Selector/4 -> [OFDM_Last_Byte]  "<last_byte>"
  DSSS data/1 -> Bus Selector1/1
  OFDM data/1 -> Bus Selector/1
  Posedge/1(Q) -> [DSSS_Byte_Valid]
  [RX_PHY_MODE_11N_AC] -> Mux/1
  Constant/1 -> Mux/2
  Constant4/1 -> Mux/3

== system wlan_phy_rx_pmd/FCS & Pkt Buf/Pkt Buf Interface ==
  DSSS data : Inport
  OFDM data : Inport  Port=2
  Addr Check : Subsystem
  BRAM IF 64b : Subsystem
  Bus Selector : BusSelector  OutputSignals=byte_valid,byte_index,byte,last_byte
  Bus Selector1 : BusSelector  OutputSignals=byte_valid,byte_index,byte,last_byte
  DSSS Addr Calc : Subsystem
  Logical7 : xbsIndex_r4/Logical  logical_function=OR  inputs=2  en=off  latency=0  precision=Full  arith_type=Unsigned  n_bits=8  bin_pt=2  align_bp=on  dbl_ovrd=off  block_type=logical
      settings: xl_area=[0, 0, 0, 0, 0, 0, 0]  block_version=VER_STRING_GOES_HERE
  Mux2 : xbsIndex_r4/Mux  inputs=2  en=off  latency=0  precision=Full  arith_type=Unsigned  n_bits=16  bin_pt=14  quantization=Truncate  overflow=Wrap  dbl_ovrd=off  block_type=mux
  Mux3 : xbsIndex_r4/Mux  inputs=2  en=off  latency=0  precision=Full  arith_type=Unsigned  n_bits=16  bin_pt=14  quantization=Truncate  overflow=Wrap  dbl_ovrd=off  block_type=mux
  Mux5 : xbsIndex_r4/Mux  inputs=2  en=off  latency=0  precision=Full  arith_type=Unsigned  n_bits=16  bin_pt=14  quantization=Truncate  overflow=Wrap  dbl_ovrd=off  block_type=mux
  OFDM Addr Calc : Subsystem
  Register{4..5,7..8} (4 alike) : xbsIndex_r4/Register  init=0  rst=off  en=off  dbl_ovrd=off  block_type=register
  Rx Data MAC Outputs : Subsystem
  wires:
  [DSSS_Byte_Valid] -> Mux3/1
  [DSSS_Byte_Index] -> DSSS Addr Calc/1(R)
  DSSS Addr Calc/1(M) -> Mux5/3
  [DSSS_Byte_Valid] -> Mux5/1
  Mux5/1 -> Addr Check/2(Addr)
  [OFDM_Byte_Index] -> OFDM Addr Calc/1(R)
  OFDM Addr Calc/1(M) -> Mux5/2
  Mux3/1 -> Register4/1
  Register4/1 -> BRAM IF 64b/4(Pkt Buf Sel)
  [CUR_RX_BYTE] -> Register7/1
  [CUR_RX_BYTE_VALID] -> Addr Check/1(WrEn)
  Register7/1 -> BRAM IF 64b/1("byte "), Rx Data MAC Outputs/1("Byte ")
  Register8/1 -> BRAM IF 64b/2(byte_valid), Rx Data MAC Outputs/2(Byte Valid)
  Register5/1 -> BRAM IF 64b/3(byte_ind), Rx Data MAC Outputs/3(Byte Ind)
  Bus Selector1/1 -> [DSSS_Byte_Valid]  "<byte_valid>"
  Bus Selector1/2 -> [DSSS_Byte_Index]  "<byte_index>"
  Bus Selector1/3 -> [DSSS_Byte]  "<byte>"
  Bus Selector1/4 -> [DSSS_Last_Byte]  "<last_byte>"
  Bus Selector/1 -> [OFDM_Byte_Valid]  "<byte_valid>"
  Bus Selector/2 -> [OFDM_Byte_Index]  "<byte_index>"
  Bus Selector/3 -> [OFDM_Byte]  "<byte>"
  Bus Selector/4 -> [OFDM_Last_Byte]  "<last_byte>"
  DSSS data/1 -> Bus Selector1/1
  OFDM data/1 -> Bus Selector/1
  [DSSS_Byte_Valid] -> Mux2/1
  [OFDM_Byte] -> Mux2/2
  [DSSS_Byte] -> Mux2/3
  Mux2/1 -> [CUR_RX_BYTE]
  [DSSS_Byte_Valid] -> Logical7/1
  [OFDM_Byte_Valid] -> Logical7/2
  Logical7/1 -> [CUR_RX_BYTE_VALID]
  [regRx_OFDM_PKT_BUF_SEL] -> Mux3/2
  [regRx_DSSS_PKT_BUF_SEL] -> Mux3/3
  Addr Check/1(" WrEn") -> Register8/1
  Addr Check/2(" Addr") -> Register5/1

== system wlan_phy_rx_pmd/FFT/Reset Gen ==
  note: Extend FFT reset for duration of TVALID + 4 to handle
      | case of pkt ending while new FFT frame is being loaded.
      | Turns out reset inputs for FIFO and FFT do not actually
      | flush internal state - both cores keep respnding to inputs
      | while reset is asserted (very frustrating...)
  FFT Valid In : Inport
  Reset : Inport  Port=2
  Inverter : xbsIndex_r4/Inverter  en=off  latency=0  dbl_ovrd=off  block_type=inv
  Inverter1 : xbsIndex_r4/Inverter  en=off  latency=4  dbl_ovrd=off  block_type=inv
  Logical : xbsIndex_r4/Logical  logical_function=AND  inputs=2  en=off  latency=0  precision=Full  arith_type=Unsigned  n_bits=16  bin_pt=0  align_bp=on  dbl_ovrd=off  block_type=logical
  Logical1 : xbsIndex_r4/Logical  logical_function=OR  inputs=2  en=off  latency=0  precision=Full  arith_type=Unsigned  n_bits=16  bin_pt=0  align_bp=on  dbl_ovrd=off  block_type=logical
  Register2 : xbsIndex_r4/Register  init=1  rst=off  en=off  dbl_ovrd=off  block_type=register
      settings: xl_area=[1 1 0 0 0 0 0]  block_version=VER_STRING_GOES_HERE
  S-R Latch1 : Subsystem
  FFT Reset : Outport
  " Reset" : Outport  Port=2
  wires:
  Inverter1/1 -> S-R Latch1/2(R)
  Reset/1 -> Logical1/1, Logical/2
  Logical/1 -> S-R Latch1/1(S)
  S-R Latch1/1(Q) -> Logical1/2
  Logical1/1 -> " Reset"/1, Inverter/1
  FFT Valid In/1 -> Inverter1/1, Logical/1
  Inverter/1 -> Register2/1
  Register2/1 -> FFT Reset/1

== system wlan_phy_rx_pmd/FFT/Sym Count ==
  note: "Symbol index" counts OFDM symbols and increments with each FFT.
      | Content of each OFDM sybmol depends on PHY mode (ag, n, or ac)
  data_in_tvalid : Inport
  pkt_done : Inport  Port=2
  data_out_tlast : Inport  Port=3
  Logical : xbsIndex_r4/Logical  logical_function=AND  inputs=2  en=off  latency=0  precision=Full  arith_type=Unsigned  n_bits=16  bin_pt=0  align_bp=on  dbl_ovrd=off  block_type=logical
  S-R Latch1 : Subsystem
  Symbol Counter : xbsIndex_r4/Counter  cnt_type=Free Running  cnt_to=Inf  operation=Up  start_count=0  cnt_by_val=1  arith_type=Unsigned  n_bits=ceil(log2(MAX_NUM_SYMS))  bin_pt=0  load_pin=off  rst=on  en=on  explicit_period=off  period=1  dbl_ovrd=off  use_behavioral_HDL=off  implementation=Fabric  block_type=counter
  Sym Ind : Outport
  wires:
  data_out_tlast/1 -> Logical/1
  Logical/1 -> Symbol Counter/2
  S-R Latch1/1(Q) -> ?
  data_in_tvalid/1 -> S-R Latch1/1(S), Logical/2
  Symbol Counter/1 -> Sym Ind/1
  pkt_done/1 -> S-R Latch1/2(R), Symbol Counter/1

== system wlan_phy_rx_pmd/Inputs/RF A Inputs ==
  Rst : Inport
  IQ Valid : Inport  Port=2
  Complex to Real-Imag : ComplexToRealImag
  Constant1 : Constant  Value=0
  Constant2 : Constant  Value=2
  Constant4 : Constant  Value=5
  Delay1 : xbsIndex_r4/Delay  rst=off  infoeditControl=Selection of Reset will increase slice count due to use of real FFs and instead of SRLs  en=off  latency=1  dbl_ovrd=off  reg_retiming=off  block_type=delay
  Delay2 : xbsIndex_r4/Delay  rst=off  infoeditControl=Selection of Reset will increase slice count due to use of real FFs and instead of SRLs  en=off  latency=1  dbl_ovrd=off  reg_retiming=off  block_type=delay
  Down Sample : xbsIndex_r4/Down Sample  sample_ratio=16  sample_phase="Last Value of Frame  (most efficient)"  en=off  latency=1  dbl_ovrd=off  block_type=dsamp
  From Workspace : FromWorkspace  VariableName=rx_sim.waveform_RFA  SampleTime=160 / rx_sim.samp_rate  Interpolate=off  OutputAfterFinalValue=Setting to zero
  RF A RSSI Sum : Subsystem
  RFA_G_BB : xbsIndex_r4/Gateway In  gui_display_data_type=Fixed-point  arith_type=Unsigned  n_bits=5  bin_pt=0  preci_type=Single  exp_width=8  frac_width=24  quantization="Round  (unbiased: +/- Inf)"  overflow=Saturate  period=1  dbl_ovrd=off  timing_constraint=None  locs_specified=off  LOCs={}  UseAsADC=off  ADCChannel='1'  inherit_from_input=off  hdl_port=on  block_type=gatewayin
  RFA_G_RF : xbsIndex_r4/Gateway In  gui_display_data_type=Fixed-point  arith_type=Unsigned  n_bits=2  bin_pt=0  preci_type=Single  exp_width=8  frac_width=24  quantization="Round  (unbiased: +/- Inf)"  overflow=Saturate  period=1  dbl_ovrd=off  timing_constraint=None  locs_specified=off  LOCs={}  UseAsADC=off  ADCChannel='1'  inherit_from_input=off  hdl_port=on  block_type=gatewayin
  RFA_RSSI : xbsIndex_r4/Gateway In  gui_display_data_type=Fixed-point  arith_type=Unsigned  n_bits=10  bin_pt=0  preci_type=Single  exp_width=8  frac_width=24  quantization="Round  (unbiased: +/- Inf)"  overflow=Saturate  period=1  dbl_ovrd=off  timing_constraint=None  locs_specified=off  LOCs={}  UseAsADC=off  ADCChannel='1'  inherit_from_input=off  hdl_port=on  block_type=gatewayin
  RFA_RX_I : xbsIndex_r4/Gateway In  gui_display_data_type=Fixed-point  arith_type="Signed  (2's comp)"  n_bits=16  bin_pt=15  preci_type=Single  exp_width=8  frac_width=24  quantization="Round  (unbiased: +/- Inf)"  overflow=Saturate  period=1  dbl_ovrd=off  timing_constraint=None  locs_specified=off  LOCs={}  UseAsADC=off  ADCChannel='1'  inherit_from_input=off  hdl_port=on  block_type=gatewayin
  RFA_RX_Q : xbsIndex_r4/Gateway In  gui_display_data_type=Fixed-point  arith_type="Signed  (2's comp)"  n_bits=16  bin_pt=15  preci_type=Single  exp_width=8  frac_width=24  quantization="Round  (unbiased: +/- Inf)"  overflow=Saturate  period=1  dbl_ovrd=off  timing_constraint=None  locs_specified=off  LOCs={}  UseAsADC=off  ADCChannel='1'  inherit_from_input=off  hdl_port=on  block_type=gatewayin
  Register : xbsIndex_r4/Register  init=0  rst=on  en=on  dbl_ovrd=off  block_type=register
  Register1 : xbsIndex_r4/Register  init=0  rst=on  en=on  dbl_ovrd=off  block_type=register
  Register2 : xbsIndex_r4/Register  init=0  rst=off  en=off  dbl_ovrd=off  block_type=register
  Register3 : xbsIndex_r4/Register  init=0  rst=on  en=off  dbl_ovrd=off  block_type=register
  Register4 : xbsIndex_r4/Register  init=0  rst=off  en=off  dbl_ovrd=off  block_type=register
  Register5 : xbsIndex_r4/Register  init=0  rst=off  en=off  dbl_ovrd=off  block_type=register
  Zero-Order Hold : ZeroOrderHold
  Zero-Order Hold1 : ZeroOrderHold
  ADC I : Outport
  ADC Q : Outport  Port=2
  Avg RSSI : Outport  Port=3
  tag scope: CS_ADC_I=global, CS_ADC_Q=global, CS_G_BB=global, CS_G_RF=global, CS_RFA_RSSI=global, RFA_AGC_G_BB=global, RFA_AGC_G_RF=global
  wires:
  Complex to Real-Imag/1 -> Zero-Order Hold/1
  Complex to Real-Imag/2 -> Zero-Order Hold1/1
  RFA_RX_I/1 -> Register2/1
  RFA_RX_Q/1 -> Register4/1
  Register/1 -> ADC I/1
  Register1/1 -> ADC Q/1
  Register3/1 -> Down Sample/1
  Down Sample/1 -> RF A RSSI Sum/1(RSSI)
  RF A RSSI Sum/1(Sum) -> [CS_RFA_RSSI], Avg RSSI/1
  RFA_RSSI/1 -> Register5/1
  Register2/1 -> Register/1, [CS_ADC_I]
  Register4/1 -> Register1/1, [CS_ADC_Q]
  Register5/1 -> Register3/1
  Rst/1 -> Register/2, Register1/2, Register3/2
  IQ Valid/1 -> Register1/3, Register/3
  RFA_G_RF/1 -> Delay1/1
  RFA_G_BB/1 -> Delay2/1
  Delay1/1 -> [CS_G_RF], [RFA_AGC_G_RF]
  Delay2/1 -> [CS_G_BB], [RFA_AGC_G_BB]
  Constant2/1 -> RFA_G_RF/1
  Constant4/1 -> RFA_G_BB/1
  Constant1/1 -> RFA_RSSI/1
  From Workspace/1 -> Complex to Real-Imag/1
  Zero-Order Hold/1 -> RFA_RX_I/1
  Zero-Order Hold1/1 -> RFA_RX_Q/1

== system wlan_phy_rx_pmd/Inputs/RF B Inputs ==
  Rst : Inport
  IQ Valid : Inport  Port=2
  Constant1 : Constant  Value=0
  Constant2 : Constant  Value=0
  Constant3 : Constant  Value=0
  Constant6 : Constant  Value=2
  Constant7 : Constant  Value=5
  Delay4 : xbsIndex_r4/Delay  rst=off  infoeditControl=Selection of Reset will increase slice count due to use of real FFs and instead of SRLs  en=off  latency=1  dbl_ovrd=off  reg_retiming=off  block_type=delay
  Delay5 : xbsIndex_r4/Delay  rst=off  infoeditControl=Selection of Reset will increase slice count due to use of real FFs and instead of SRLs  en=off  latency=1  dbl_ovrd=off  reg_retiming=off  block_type=delay
  Down Sample : xbsIndex_r4/Down Sample  sample_ratio=16  sample_phase="Last Value of Frame  (most efficient)"  en=off  latency=1  dbl_ovrd=off  block_type=dsamp
  RF B RSSI Sum : Subsystem
  RFB_G_BB : xbsIndex_r4/Gateway In  gui_display_data_type=Fixed-point  arith_type=Unsigned  n_bits=5  bin_pt=0  preci_type=Single  exp_width=8  frac_width=24  quantization="Round  (unbiased: +/- Inf)"  overflow=Saturate  period=1  dbl_ovrd=off  timing_constraint=None  locs_specified=off  LOCs={}  UseAsADC=off  ADCChannel='1'  inherit_from_input=off  hdl_port=on  block_type=gatewayin
  RFB_G_RF : xbsIndex_r4/Gateway In  gui_display_data_type=Fixed-point  arith_type=Unsigned  n_bits=2  bin_pt=0  preci_type=Single  exp_width=8  frac_width=24  quantization="Round  (unbiased: +/- Inf)"  overflow=Saturate  period=1  dbl_ovrd=off  timing_constraint=None  locs_specified=off  LOCs={}  UseAsADC=off  ADCChannel='1'  inherit_from_input=off  hdl_port=on  block_type=gatewayin
  RFB_RSSI : xbsIndex_r4/Gateway In  gui_display_data_type=Fixed-point  arith_type=Unsigned  n_bits=10  bin_pt=0  preci_type=Single  exp_width=8  frac_width=24  quantization="Round  (unbiased: +/- Inf)"  overflow=Saturate  period=1  dbl_ovrd=off  timing_constraint=None  locs_specified=off  LOCs={}  UseAsADC=off  ADCChannel='1'  inherit_from_input=off  hdl_port=on  block_type=gatewayin
  RFB_RX_I : xbsIndex_r4/Gateway In  gui_display_data_type=Fixed-point  arith_type="Signed  (2's comp)"  n_bits=16  bin_pt=15  preci_type=Single  exp_width=8  frac_width=24  quantization="Round  (unbiased: +/- Inf)"  overflow=Saturate  period=1  dbl_ovrd=off  timing_constraint=None  locs_specified=off  LOCs={}  UseAsADC=off  ADCChannel='1'  inherit_from_input=off  hdl_port=on  block_type=gatewayin
  RFB_RX_Q : xbsIndex_r4/Gateway In  gui_display_data_type=Fixed-point  arith_type="Signed  (2's comp)"  n_bits=16  bin_pt=15  preci_type=Single  exp_width=8  frac_width=24  quantization="Round  (unbiased: +/- Inf)"  overflow=Saturate  period=1  dbl_ovrd=off  timing_constraint=None  locs_specified=off  LOCs={}  UseAsADC=off  ADCChannel='1'  inherit_from_input=off  hdl_port=on  block_type=gatewayin
  Register : xbsIndex_r4/Register  init=0  rst=on  en=on  dbl_ovrd=off  block_type=register
  Register1 : xbsIndex_r4/Register  init=0  rst=on  en=on  dbl_ovrd=off  block_type=register
  Register2 : xbsIndex_r4/Register  init=0  rst=off  en=off  dbl_ovrd=off  block_type=register
  Register3 : xbsIndex_r4/Register  init=0  rst=on  en=off  dbl_ovrd=off  block_type=register
  Register4 : xbsIndex_r4/Register  init=0  rst=off  en=off  dbl_ovrd=off  block_type=register
  Register5 : xbsIndex_r4/Register  init=0  rst=off  en=off  dbl_ovrd=off  block_type=register
  ADC I : Outport
  ADC Q : Outport  Port=2
  Avg RSSI : Outport  Port=3
  tag scope: RFB_AGC_G_BB=global, RFB_AGC_G_RF=global
  wires:
  IQ Valid/1 -> Register/3, Register1/3
  Rst/1 -> Register3/2, Register1/2, Register/2
  Register5/1 -> Register3/1
  Register4/1 -> Register1/1
  Register2/1 -> Register/1
  RFB_RSSI/1 -> Register5/1
  RF B RSSI Sum/1(Sum) -> Avg RSSI/1
  Down Sample/1 -> RF B RSSI Sum/1(RSSI)
  Register3/1 -> Down Sample/1
  Register1/1 -> ADC Q/1
  Register/1 -> ADC I/1
  RFB_RX_Q/1 -> Register4/1
  RFB_RX_I/1 -> Register2/1
  RFB_G_RF/1 -> Delay4/1
  RFB_G_BB/1 -> Delay5/1
  Delay4/1 -> [RFB_AGC_G_RF]
  Delay5/1 -> [RFB_AGC_G_BB]
  Constant6/1 -> RFB_G_RF/1
  Constant7/1 -> RFB_G_BB/1
  Constant1/1 -> RFB_RX_I/1
  Constant3/1 -> RFB_RSSI/1
  Constant2/1 -> RFB_RX_Q/1

== system wlan_phy_rx_pmd/Inputs/RF C Inputs ==
  Rst : Inport
  IQ Valid : Inport  Port=2
  Constant1 : Constant  Value=0
  Constant2 : Constant  Value=2
  Constant3 : Constant  Value=0
  Constant4 : Constant  Value=5
  Constant5 : Constant  Value=0
  Delay1 : xbsIndex_r4/Delay  rst=off  infoeditControl=Selection of Reset will increase slice count due to use of real FFs and instead of SRLs  en=off  latency=1  dbl_ovrd=off  reg_retiming=off  block_type=delay
  Delay2 : xbsIndex_r4/Delay  rst=off  infoeditControl=Selection of Reset will increase slice count due to use of real FFs and instead of SRLs  en=off  latency=1  dbl_ovrd=off  reg_retiming=off  block_type=delay
  Down Sample : xbsIndex_r4/Down Sample  sample_ratio=16  sample_phase="Last Value of Frame  (most efficient)"  en=off  latency=1  dbl_ovrd=off  block_type=dsamp
  RF C RSSI Sum : Subsystem
  RFC_G_BB : xbsIndex_r4/Gateway In  gui_display_data_type=Fixed-point  arith_type=Unsigned  n_bits=5  bin_pt=0  preci_type=Single  exp_width=8  frac_width=24  quantization="Round  (unbiased: +/- Inf)"  overflow=Saturate  period=1  dbl_ovrd=off  timing_constraint=None  locs_specified=off  LOCs={}  UseAsADC=off  ADCChannel='1'  inherit_from_input=off  hdl_port=on  block_type=gatewayin
  RFC_G_RF : xbsIndex_r4/Gateway In  gui_display_data_type=Fixed-point  arith_type=Unsigned  n_bits=2  bin_pt=0  preci_type=Single  exp_width=8  frac_width=24  quantization="Round  (unbiased: +/- Inf)"  overflow=Saturate  period=1  dbl_ovrd=off  timing_constraint=None  locs_specified=off  LOCs={}  UseAsADC=off  ADCChannel='1'  inherit_from_input=off  hdl_port=on  block_type=gatewayin
  RFC_RSSI : xbsIndex_r4/Gateway In  gui_display_data_type=Fixed-point  arith_type=Unsigned  n_bits=10  bin_pt=0  preci_type=Single  exp_width=8  frac_width=24  quantization="Round  (unbiased: +/- Inf)"  overflow=Saturate  period=1  dbl_ovrd=off  timing_constraint=None  locs_specified=off  LOCs={}  UseAsADC=off  ADCChannel='1'  inherit_from_input=off  hdl_port=on  block_type=gatewayin
  RFC_RX_I : xbsIndex_r4/Gateway In  gui_display_data_type=Fixed-point  arith_type="Signed  (2's comp)"  n_bits=16  bin_pt=15  preci_type=Single  exp_width=8  frac_width=24  quantization="Round  (unbiased: +/- Inf)"  overflow=Saturate  period=1  dbl_ovrd=off  timing_constraint=None  locs_specified=off  LOCs={}  UseAsADC=off  ADCChannel='1'  inherit_from_input=off  hdl_port=on  block_type=gatewayin
  RFC_RX_Q : xbsIndex_r4/Gateway In  gui_display_data_type=Fixed-point  arith_type="Signed  (2's comp)"  n_bits=16  bin_pt=15  preci_type=Single  exp_width=8  frac_width=24  quantization="Round  (unbiased: +/- Inf)"  overflow=Saturate  period=1  dbl_ovrd=off  timing_constraint=None  locs_specified=off  LOCs={}  UseAsADC=off  ADCChannel='1'  inherit_from_input=off  hdl_port=on  block_type=gatewayin
  Register : xbsIndex_r4/Register  init=0  rst=on  en=on  dbl_ovrd=off  block_type=register
  Register1 : xbsIndex_r4/Register  init=0  rst=on  en=on  dbl_ovrd=off  block_type=register
  Register2 : xbsIndex_r4/Register  init=0  rst=off  en=off  dbl_ovrd=off  block_type=register
  Register3 : xbsIndex_r4/Register  init=0  rst=on  en=off  dbl_ovrd=off  block_type=register
  Register4 : xbsIndex_r4/Register  init=0  rst=off  en=off  dbl_ovrd=off  block_type=register
  Register5 : xbsIndex_r4/Register  init=0  rst=off  en=off  dbl_ovrd=off  block_type=register
  ADC I : Outport
  ADC Q : Outport  Port=2
  Avg RSSI : Outport  Port=3
  tag scope: CS_ADC_I_RFC=global, CS_ADC_Q_RFC=global, RFC_AGC_G_BB=global, RFC_AGC_G_RF=global
  wires:
  Constant4/1 -> RFC_G_BB/1
  Constant2/1 -> RFC_G_RF/1
  Delay2/1 -> [RFC_AGC_G_BB]
  Delay1/1 -> [RFC_AGC_G_RF]
  RFC_G_BB/1 -> Delay2/1
  RFC_G_RF/1 -> Delay1/1
  IQ Valid/1 -> Register/3, Register1/3
  Rst/1 -> Register3/2, Register1/2, Register/2
  Register5/1 -> Register3/1
  Register4/1 -> Register1/1, [CS_ADC_Q_RFC]
  Register2/1 -> Register/1, [CS_ADC_I_RFC]
  RFC_RSSI/1 -> Register5/1
  RF C RSSI Sum/1(Sum) -> Avg RSSI/1
  Down Sample/1 -> RF C RSSI Sum/1(RSSI)
  Register3/1 -> Down Sample/1
  Register1/1 -> ADC Q/1
  Register/1 -> ADC I/1
  RFC_RX_Q/1 -> Register4/1
  RFC_RX_I/1 -> Register2/1
  Constant3/1 -> RFC_RX_Q/1
  Constant1/1 -> RFC_RX_I/1
  Constant5/1 -> RFC_RSSI/1
